simulink model "quantized"  (R2015b) (continued)
== system quantized == (continued)
  ri_to_c10/1 -> [ant_9]
  Convert20/1 -> Relational22/2, Mux20/2
  Relational22/1 -> Mux20/1
  Constant50/1 -> Mux20/3
  Constant43/1 -> Relational22/1
  Convert21/1 -> Relational23/2, Mux21/2
  Relational23/1 -> Mux21/1
  Constant51/1 -> Mux21/3
  Constant44/1 -> Relational23/1
  Mux20/1 -> ri_to_c11/1
  Mux21/1 -> ri_to_c11/2
  ri_to_c11/1 -> [ant_11]
  c_to_ri9/1 -> Mult22/1
  c_to_ri9/2 -> Mult23/2
  Constant52/1 -> scale8/1(sim_1)
  Mult22/1 -> Convert22/1
  Mult23/1 -> Convert23/1
  scale8/1(in_reg) -> Mult22/2, Mult23/1
  c_to_ri10/1 -> Mult16/1
  c_to_ri10/2 -> Mult17/2
  Constant53/1 -> scale10/1(sim_1)
  Mult16/1 -> Convert16/1
  Mult17/1 -> Convert17/1
  scale10/1(in_reg) -> Mult16/2, Mult17/1
  c_to_ri11/1 -> Mult18/1
  c_to_ri11/2 -> Mult19/2
  Constant54/1 -> scale9/1(sim_1)
  Mult18/1 -> Convert18/1
  Mult19/1 -> Convert19/1
  scale9/1(in_reg) -> Mult18/2, Mult19/1
  c_to_ri12/1 -> Mult20/1
  c_to_ri12/2 -> Mult21/2
  Constant55/1 -> scale11/1(sim_1)
  Mult20/1 -> Convert20/1
  Mult21/1 -> Convert21/1
  scale11/1(in_reg) -> Mult20/2, Mult21/1
  Register/1 -> c_to_ri1/1
  Register1/1 -> c_to_ri2/1
  Register2/1 -> c_to_ri3/1
  Register3/1 -> c_to_ri4/1
  Register4/1 -> c_to_ri5/1
  Register5/1 -> c_to_ri6/1
  Register6/1 -> c_to_ri7/1
  Register7/1 -> c_to_ri8/1
  Register8/1 -> c_to_ri9/1
  Register9/1 -> c_to_ri10/1
  Register10/1 -> c_to_ri11/1
  Register11/1 -> c_to_ri12/1
  addr_bram_vacc4/1(addr) -> ac_a4_real/1(addr)
  addr_bram_vacc4/2(dout) -> ac_a4_real/2(data_in)
  addr_bram_vacc4/3(we) -> ac_a4_real/3(we)
  ac_a4_real/1(data_out) -> Terminator8/1
  addr_bram_vacc5/1(addr) -> ac_a5_real/1(addr)
  addr_bram_vacc5/2(dout) -> ac_a5_real/2(data_in)
  addr_bram_vacc5/3(we) -> ac_a5_real/3(we)
  ac_a5_real/1(data_out) -> Terminator10/1
  addr_bram_vacc6/1(addr) -> ac_a6_real/1(addr)
  addr_bram_vacc6/2(dout) -> ac_a6_real/2(data_in)
  addr_bram_vacc6/3(we) -> ac_a6_real/3(we)
  ac_a6_real/1(data_out) -> Terminator7/1
  addr_bram_vacc7/1(addr) -> ac_a7_real/1(addr)
  addr_bram_vacc7/2(dout) -> ac_a7_real/2(data_in)
  addr_bram_vacc7/3(we) -> ac_a7_real/3(we)
  ac_a7_real/1(data_out) -> Terminator4/1
  [ant_4] -> cmult5/2(b), cmult5/1(a)
  [ant_5] -> cmult6/2(b), cmult6/1(a)
  [ant_6] -> cmult7/2(b), cmult7/1(a)
  [ant_7] -> cmult8/2(b), cmult8/1(a)
  Slice5/1 -> Reinterpret4/1
  Reinterpret4/1 -> addr_bram_vacc4/2(din)
  cmult5/1(ab) -> Slice5/1
  Slice6/1 -> Reinterpret5/1
  Reinterpret5/1 -> addr_bram_vacc5/2(din)
  cmult6/1(ab) -> Slice6/1
  Slice8/1 -> Reinterpret7/1
  Slice7/1 -> Reinterpret6/1
  Reinterpret6/1 -> addr_bram_vacc6/2(din)
  Reinterpret7/1 -> addr_bram_vacc7/2(din)
  cmult7/1(ab) -> Slice7/1
  cmult8/1(ab) -> Slice8/1
  addr_bram_vacc8/1(addr) -> ac_a8_real/1(addr)
  addr_bram_vacc8/2(dout) -> ac_a8_real/2(data_in)
  addr_bram_vacc8/3(we) -> ac_a8_real/3(we)
  ac_a8_real/1(data_out) -> Terminator14/1
  addr_bram_vacc9/1(addr) -> ac_a9_real/1(addr)
  addr_bram_vacc9/2(dout) -> ac_a9_real/2(data_in)
  addr_bram_vacc9/3(we) -> ac_a9_real/3(we)
  ac_a9_real/1(data_out) -> Terminator15/1
  [new_acc] -> addr_bram_vacc11/1(new_acc), addr_bram_vacc10/1(new_acc), addr_bram_vacc9/1(new_acc), addr_bram_vacc8/1(new_acc), addr_bram_vacc7/1(new_acc), addr_bram_vacc6/1(new_acc), addr_bram_vacc5/1(new_acc), addr_bram_vacc4/1(new_acc), addr_bram_vacc3/1(new_acc), addr_bram_vacc2/1(new_acc), addr_bram_vacc1/1(new_acc), addr_bram_vacc/1(new_acc)
  addr_bram_vacc10/1(addr) -> ac_a10_real/1(addr)
  addr_bram_vacc10/2(dout) -> ac_a10_real/2(data_in)
  addr_bram_vacc10/3(we) -> ac_a10_real/3(we)
  ac_a10_real/1(data_out) -> Terminator13/1
  addr_bram_vacc11/1(addr) -> ac_a11_real/1(addr)
  addr_bram_vacc11/2(dout) -> ac_a11_real/2(data_in)
  addr_bram_vacc11/3(we) -> ac_a11_real/3(we)
  ac_a11_real/1(data_out) -> Terminator12/1
  [ant_8] -> cmult9/2(b), cmult9/1(a)
  [ant_9] -> cmult10/2(b), cmult10/1(a)
  [ant_10] -> cmult11/2(b), cmult11/1(a)
  [ant_11] -> cmult12/2(b), cmult12/1(a)
  Slice9/1 -> Reinterpret8/1
  Reinterpret8/1 -> addr_bram_vacc8/2(din)
  cmult9/1(ab) -> Slice9/1
  Slice10/1 -> Reinterpret9/1
  Reinterpret9/1 -> addr_bram_vacc9/2(din)
  cmult10/1(ab) -> Slice10/1
  Slice12/1 -> Reinterpret11/1
  Slice11/1 -> Reinterpret10/1
  Reinterpret10/1 -> addr_bram_vacc10/2(din)
  Reinterpret11/1 -> addr_bram_vacc11/2(din)
  cmult11/1(ab) -> Slice11/1
  cmult12/1(ab) -> Slice12/1
  cmult/1(ab) -> c_to_ri/1
  addr_bram_vacc18/1(addr) -> cc_a0_a1_real/1(addr)
  addr_bram_vacc18/2(dout) -> cc_a0_a1_real/2(data_in)
  addr_bram_vacc18/3(we) -> cc_a0_a1_real/3(we)
  cc_a0_a1_real/1(data_out) -> Terminator26/1
  addr_bram_vacc19/1(addr) -> cc_a0_a1_imag/1(addr)
  addr_bram_vacc19/2(dout) -> cc_a0_a1_imag/2(data_in)
  addr_bram_vacc19/3(we) -> cc_a0_a1_imag/3(we)
  cc_a0_a1_imag/1(data_out) -> Terminator27/1
  c_to_ri/1 -> addr_bram_vacc18/2(din)
  c_to_ri/2 -> addr_bram_vacc19/2(din)
  cmult13/1(ab) -> c_to_ri75/1
  addr_bram_vacc20/1(addr) -> cc_a0_a2_real/1(addr)
  addr_bram_vacc20/2(dout) -> cc_a0_a2_real/2(data_in)
  addr_bram_vacc20/3(we) -> cc_a0_a2_real/3(we)
  cc_a0_a2_real/1(data_out) -> Terminator16/1
  addr_bram_vacc21/1(addr) -> cc_a0_a2_imag/1(addr)
  addr_bram_vacc21/2(dout) -> cc_a0_a2_imag/2(data_in)
  addr_bram_vacc21/3(we) -> cc_a0_a2_imag/3(we)
  cc_a0_a2_imag/1(data_out) -> Terminator17/1
  c_to_ri75/1 -> addr_bram_vacc20/2(din)
  c_to_ri75/2 -> addr_bram_vacc21/2(din)
  cmult14/1(ab) -> c_to_ri76/1
  addr_bram_vacc22/1(addr) -> cc_a0_a3_real/1(addr)
  addr_bram_vacc22/2(dout) -> cc_a0_a3_real/2(data_in)
  addr_bram_vacc22/3(we) -> cc_a0_a3_real/3(we)
  cc_a0_a3_real/1(data_out) -> Terminator18/1
  addr_bram_vacc23/1(addr) -> cc_a0_a3_imag/1(addr)
  addr_bram_vacc23/2(dout) -> cc_a0_a3_imag/2(data_in)
  addr_bram_vacc23/3(we) -> cc_a0_a3_imag/3(we)
  cc_a0_a3_imag/1(data_out) -> Terminator19/1
  c_to_ri76/1 -> addr_bram_vacc22/2(din)
  c_to_ri76/2 -> addr_bram_vacc23/2(din)
  cmult15/1(ab) -> c_to_ri77/1
  addr_bram_vacc12/1(addr) -> cc_a1_a2_real/1(addr)
  addr_bram_vacc12/2(dout) -> cc_a1_a2_real/2(data_in)
  addr_bram_vacc12/3(we) -> cc_a1_a2_real/3(we)
  cc_a1_a2_real/1(data_out) -> Terminator20/1
  addr_bram_vacc13/1(addr) -> cc_a1_a2_imag/1(addr)
  addr_bram_vacc13/2(dout) -> cc_a1_a2_imag/2(data_in)
  addr_bram_vacc13/3(we) -> cc_a1_a2_imag/3(we)
  cc_a1_a2_imag/1(data_out) -> Terminator21/1
  c_to_ri77/1 -> addr_bram_vacc12/2(din)
  c_to_ri77/2 -> addr_bram_vacc13/2(din)
  cmult16/1(ab) -> c_to_ri14/1
  addr_bram_vacc14/1(addr) -> cc_a1_a3_real/1(addr)
  addr_bram_vacc14/2(dout) -> cc_a1_a3_real/2(data_in)
  addr_bram_vacc14/3(we) -> cc_a1_a3_real/3(we)
  cc_a1_a3_real/1(data_out) -> Terminator22/1
  addr_bram_vacc15/1(addr) -> cc_a1_a3_imag/1(addr)
  addr_bram_vacc15/2(dout) -> cc_a1_a3_imag/2(data_in)
  addr_bram_vacc15/3(we) -> cc_a1_a3_imag/3(we)
  cc_a1_a3_imag/1(data_out) -> Terminator23/1
  c_to_ri14/1 -> addr_bram_vacc14/2(din)
  c_to_ri14/2 -> addr_bram_vacc15/2(din)
  [ant_0] -> cmult/1(a)
  [ant_1] -> cmult/2(b)
  [ant_0] -> cmult13/1(a)
  [ant_2] -> cmult13/2(b)
  [ant_3] -> cmult14/2(b)
  [ant_0] -> cmult14/1(a)
  [ant_2] -> cmult15/2(b)
  [ant_1] -> cmult15/1(a)
  [ant_3] -> cmult16/2(b)
  [ant_1] -> cmult16/1(a)
  cmult41/1(ab) -> c_to_ri36/1
  addr_bram_vacc16/1(addr) -> cc_a2_a3_real/1(addr)
  addr_bram_vacc16/2(dout) -> cc_a2_a3_real/2(data_in)
  addr_bram_vacc16/3(we) -> cc_a2_a3_real/3(we)
  cc_a2_a3_real/1(data_out) -> Terminator24/1
  addr_bram_vacc17/1(addr) -> cc_a2_a3_imag/1(addr)
  addr_bram_vacc17/2(dout) -> cc_a2_a3_imag/2(data_in)
  addr_bram_vacc17/3(we) -> cc_a2_a3_imag/3(we)
  cc_a2_a3_imag/1(data_out) -> Terminator25/1
  c_to_ri36/1 -> addr_bram_vacc16/2(din)
  c_to_ri36/2 -> addr_bram_vacc17/2(din)
  [ant_3] -> cmult41/2(b)
  [ant_2] -> cmult41/1(a)
  cmult17/1(ab) -> c_to_ri13/1
  addr_bram_vacc24/1(addr) -> cc_a0_a4_real/1(addr)
  addr_bram_vacc24/2(dout) -> cc_a0_a4_real/2(data_in)
  addr_bram_vacc24/3(we) -> cc_a0_a4_real/3(we)
  cc_a0_a4_real/1(data_out) -> Terminator32/1
  addr_bram_vacc25/1(addr) -> cc_a0_a4_imag/1(addr)
  addr_bram_vacc25/2(dout) -> cc_a0_a4_imag/2(data_in)
  addr_bram_vacc25/3(we) -> cc_a0_a4_imag/3(we)
  cc_a0_a4_imag/1(data_out) -> Terminator33/1
  c_to_ri13/1 -> addr_bram_vacc24/2(din)
  c_to_ri13/2 -> addr_bram_vacc25/2(din)
  cmult18/1(ab) -> c_to_ri24/1
  addr_bram_vacc26/1(addr) -> cc_a0_a5_real/1(addr)
  addr_bram_vacc26/2(dout) -> cc_a0_a5_real/2(data_in)
  addr_bram_vacc26/3(we) -> cc_a0_a5_real/3(we)
  cc_a0_a5_real/1(data_out) -> Terminator28/1
  addr_bram_vacc27/1(addr) -> cc_a0_a5_imag/1(addr)
  addr_bram_vacc27/2(dout) -> cc_a0_a5_imag/2(data_in)
  addr_bram_vacc27/3(we) -> cc_a0_a5_imag/3(we)
  cc_a0_a5_imag/1(data_out) -> Terminator29/1
  c_to_ri24/1 -> addr_bram_vacc26/2(din)
  c_to_ri24/2 -> addr_bram_vacc27/2(din)
  cmult19/1(ab) -> c_to_ri35/1
  addr_bram_vacc28/1(addr) -> cc_a0_a6_real/1(addr)
  addr_bram_vacc28/2(dout) -> cc_a0_a6_real/2(data_in)
  addr_bram_vacc28/3(we) -> cc_a0_a6_real/3(we)
  cc_a0_a6_real/1(data_out) -> Terminator30/1
  addr_bram_vacc29/1(addr) -> cc_a0_a6_imag/1(addr)
  addr_bram_vacc29/2(dout) -> cc_a0_a6_imag/2(data_in)
  addr_bram_vacc29/3(we) -> cc_a0_a6_imag/3(we)
  cc_a0_a6_imag/1(data_out) -> Terminator31/1
  c_to_ri35/1 -> addr_bram_vacc28/2(din)
  c_to_ri35/2 -> addr_bram_vacc29/2(din)
  [ant_0] -> cmult17/1(a)
  [ant_4] -> cmult17/2(b)
  [ant_0] -> cmult18/1(a)
  [ant_5] -> cmult18/2(b)
  [ant_6] -> cmult19/2(b)
  [ant_0] -> cmult19/1(a)
  cmult20/1(ab) -> c_to_ri46/1
  addr_bram_vacc30/1(addr) -> cc_a0_a7_real/1(addr)
  addr_bram_vacc30/2(dout) -> cc_a0_a7_real/2(data_in)
  addr_bram_vacc30/3(we) -> cc_a0_a7_real/3(we)
  cc_a0_a7_real/1(data_out) -> Terminator38/1
  addr_bram_vacc31/1(addr) -> cc_a0_a7_imag/1(addr)
  addr_bram_vacc31/2(dout) -> cc_a0_a7_imag/2(data_in)
  addr_bram_vacc31/3(we) -> cc_a0_a7_imag/3(we)
  cc_a0_a7_imag/1(data_out) -> Terminator39/1
  c_to_ri46/1 -> addr_bram_vacc30/2(din)
  c_to_ri46/2 -> addr_bram_vacc31/2(din)
  cmult21/1(ab) -> c_to_ri57/1
  addr_bram_vacc32/1(addr) -> cc_a0_a8_real/1(addr)
  addr_bram_vacc32/2(dout) -> cc_a0_a8_real/2(data_in)
  addr_bram_vacc32/3(we) -> cc_a0_a8_real/3(we)
  cc_a0_a8_real/1(data_out) -> Terminator34/1
  addr_bram_vacc33/1(addr) -> cc_a0_a8_imag/1(addr)
  addr_bram_vacc33/2(dout) -> cc_a0_a8_imag/2(data_in)
  addr_bram_vacc33/3(we) -> cc_a0_a8_imag/3(we)
  cc_a0_a8_imag/1(data_out) -> Terminator35/1
  c_to_ri57/1 -> addr_bram_vacc32/2(din)
  c_to_ri57/2 -> addr_bram_vacc33/2(din)
  cmult22/1(ab) -> c_to_ri68/1
  addr_bram_vacc34/1(addr) -> cc_a0_a9_real/1(addr)
  addr_bram_vacc34/2(dout) -> cc_a0_a9_real/2(data_in)
  addr_bram_vacc34/3(we) -> cc_a0_a9_real/3(we)
  cc_a0_a9_real/1(data_out) -> Terminator36/1
  addr_bram_vacc35/1(addr) -> cc_a0_a9_imag/1(addr)
  addr_bram_vacc35/2(dout) -> cc_a0_a9_imag/2(data_in)
  addr_bram_vacc35/3(we) -> cc_a0_a9_imag/3(we)
  cc_a0_a9_imag/1(data_out) -> Terminator37/1
  c_to_ri68/1 -> addr_bram_vacc34/2(din)
  c_to_ri68/2 -> addr_bram_vacc35/2(din)
  [ant_0] -> cmult20/1(a)
  [ant_7] -> cmult20/2(b)
  [ant_0] -> cmult21/1(a)
  [ant_8] -> cmult21/2(b)
  [ant_9] -> cmult22/2(b)
  [ant_0] -> cmult22/1(a)
  cmult23/1(ab) -> c_to_ri15/1
  addr_bram_vacc36/1(addr) -> cc_a0_a10_real/1(addr)
  addr_bram_vacc36/2(dout) -> cc_a0_a10_real/2(data_in)
  addr_bram_vacc36/3(we) -> cc_a0_a10_real/3(we)
  cc_a0_a10_real/1(data_out) -> Terminator44/1
  addr_bram_vacc37/1(addr) -> cc_a0_a10_imag/1(addr)
  addr_bram_vacc37/2(dout) -> cc_a0_a10_imag/2(data_in)
  addr_bram_vacc37/3(we) -> cc_a0_a10_imag/3(we)
  cc_a0_a10_imag/1(data_out) -> Terminator45/1
  c_to_ri15/1 -> addr_bram_vacc36/2(din)
  c_to_ri15/2 -> addr_bram_vacc37/2(din)
  cmult24/1(ab) -> c_to_ri16/1
  addr_bram_vacc38/1(addr) -> cc_a0_a11_real/1(addr)
  addr_bram_vacc38/2(dout) -> cc_a0_a11_real/2(data_in)
  addr_bram_vacc38/3(we) -> cc_a0_a11_real/3(we)
  cc_a0_a11_real/1(data_out) -> Terminator40/1
  addr_bram_vacc39/1(addr) -> cc_a0_a11_imag/1(addr)
  addr_bram_vacc39/2(dout) -> cc_a0_a11_imag/2(data_in)
  addr_bram_vacc39/3(we) -> cc_a0_a11_imag/3(we)
  cc_a0_a11_imag/1(data_out) -> Terminator41/1
  c_to_ri16/1 -> addr_bram_vacc38/2(din)
  c_to_ri16/2 -> addr_bram_vacc39/2(din)
  [ant_0] -> cmult23/1(a)
  [ant_10] -> cmult23/2(b)
  [ant_0] -> cmult24/1(a)
  [ant_11] -> cmult24/2(b)
  [new_acc] -> addr_bram_vacc19/1(new_acc), addr_bram_vacc20/1(new_acc), addr_bram_vacc23/1(new_acc), addr_bram_vacc24/1(new_acc), addr_bram_vacc25/1(new_acc), addr_bram_vacc26/1(new_acc), addr_bram_vacc30/1(new_acc), addr_bram_vacc31/1(new_acc), addr_bram_vacc32/1(new_acc), addr_bram_vacc35/1(new_acc), addr_bram_vacc36/1(new_acc), addr_bram_vacc37/1(new_acc), addr_bram_vacc38/1(new_acc), addr_bram_vacc39/1(new_acc), addr_bram_vacc34/1(new_acc), addr_bram_vacc33/1(new_acc), addr_bram_vacc29/1(new_acc), addr_bram_vacc28/1(new_acc), addr_bram_vacc27/1(new_acc), addr_bram_vacc22/1(new_acc), addr_bram_vacc21/1(new_acc), addr_bram_vacc18/1(new_acc)
  cmult25/1(ab) -> c_to_ri18/1
  addr_bram_vacc40/1(addr) -> cc_a1_a4_real/1(addr)
  addr_bram_vacc40/2(dout) -> cc_a1_a4_real/2(data_in)
  addr_bram_vacc40/3(we) -> cc_a1_a4_real/3(we)
  cc_a1_a4_real/1(data_out) -> Terminator42/1
  addr_bram_vacc41/1(addr) -> cc_a1_a4_imag/1(addr)
  addr_bram_vacc41/2(dout) -> cc_a1_a4_imag/2(data_in)
  addr_bram_vacc41/3(we) -> cc_a1_a4_imag/3(we)
  cc_a1_a4_imag/1(data_out) -> Terminator43/1
  c_to_ri18/1 -> addr_bram_vacc40/2(din)
  c_to_ri18/2 -> addr_bram_vacc41/2(din)
  cmult26/1(ab) -> c_to_ri17/1
  addr_bram_vacc42/1(addr) -> cc_a1_a5_real/1(addr)
  addr_bram_vacc42/2(dout) -> cc_a1_a5_real/2(data_in)
  addr_bram_vacc42/3(we) -> cc_a1_a5_real/3(we)
  cc_a1_a5_real/1(data_out) -> Terminator46/1
  addr_bram_vacc43/1(addr) -> cc_a1_a5_imag/1(addr)
  addr_bram_vacc43/2(dout) -> cc_a1_a5_imag/2(data_in)
  addr_bram_vacc43/3(we) -> cc_a1_a5_imag/3(we)
  cc_a1_a5_imag/1(data_out) -> Terminator47/1
  c_to_ri17/1 -> addr_bram_vacc42/2(din)
  c_to_ri17/2 -> addr_bram_vacc43/2(din)
  [ant_4] -> cmult25/2(b)
  [ant_1] -> cmult25/1(a)
  [ant_5] -> cmult26/2(b)
  [ant_1] -> cmult26/1(a)
  cmult27/1(ab) -> c_to_ri20/1
  addr_bram_vacc44/1(addr) -> cc_a1_a6_real/1(addr)
  addr_bram_vacc44/2(dout) -> cc_a1_a6_real/2(data_in)
  addr_bram_vacc44/3(we) -> cc_a1_a6_real/3(we)
  cc_a1_a6_real/1(data_out) -> Terminator48/1
  addr_bram_vacc45/1(addr) -> cc_a1_a6_imag/1(addr)
  addr_bram_vacc45/2(dout) -> cc_a1_a6_imag/2(data_in)
  addr_bram_vacc45/3(we) -> cc_a1_a6_imag/3(we)
  cc_a1_a6_imag/1(data_out) -> Terminator49/1
  c_to_ri20/1 -> addr_bram_vacc44/2(din)
  c_to_ri20/2 -> addr_bram_vacc45/2(din)
  cmult28/1(ab) -> c_to_ri19/1
  addr_bram_vacc46/1(addr) -> cc_a1_a7_real/1(addr)
  addr_bram_vacc46/2(dout) -> cc_a1_a7_real/2(data_in)
  addr_bram_vacc46/3(we) -> cc_a1_a7_real/3(we)
  cc_a1_a7_real/1(data_out) -> Terminator50/1
  addr_bram_vacc47/1(addr) -> cc_a1_a7_imag/1(addr)
  addr_bram_vacc47/2(dout) -> cc_a1_a7_imag/2(data_in)
  addr_bram_vacc47/3(we) -> cc_a1_a7_imag/3(we)
  cc_a1_a7_imag/1(data_out) -> Terminator51/1
  c_to_ri19/1 -> addr_bram_vacc46/2(din)
  c_to_ri19/2 -> addr_bram_vacc47/2(din)
  [ant_6] -> cmult27/2(b)
  [ant_1] -> cmult27/1(a)
  [ant_7] -> cmult28/2(b)
  [ant_1] -> cmult28/1(a)
  cmult29/1(ab) -> c_to_ri22/1
  addr_bram_vacc48/1(addr) -> cc_a1_a8_real/1(addr)
  addr_bram_vacc48/2(dout) -> cc_a1_a8_real/2(data_in)
  addr_bram_vacc48/3(we) -> cc_a1_a8_real/3(we)
  cc_a1_a8_real/1(data_out) -> Terminator52/1
  addr_bram_vacc49/1(addr) -> cc_a1_a8_imag/1(addr)
  addr_bram_vacc49/2(dout) -> cc_a1_a8_imag/2(data_in)
  addr_bram_vacc49/3(we) -> cc_a1_a8_imag/3(we)
  cc_a1_a8_imag/1(data_out) -> Terminator53/1
  c_to_ri22/1 -> addr_bram_vacc48/2(din)
  c_to_ri22/2 -> addr_bram_vacc49/2(din)
  cmult30/1(ab) -> c_to_ri21/1
  addr_bram_vacc50/1(addr) -> cc_a1_a9_real/1(addr)
  addr_bram_vacc50/2(dout) -> cc_a1_a9_real/2(data_in)
  addr_bram_vacc50/3(we) -> cc_a1_a9_real/3(we)
  cc_a1_a9_real/1(data_out) -> Terminator54/1
  addr_bram_vacc51/1(addr) -> cc_a1_a9_imag/1(addr)
  addr_bram_vacc51/2(dout) -> cc_a1_a9_imag/2(data_in)
  addr_bram_vacc51/3(we) -> cc_a1_a9_imag/3(we)
  cc_a1_a9_imag/1(data_out) -> Terminator55/1
  c_to_ri21/1 -> addr_bram_vacc50/2(din)
  c_to_ri21/2 -> addr_bram_vacc51/2(din)
  [ant_8] -> cmult29/2(b)
  [ant_1] -> cmult29/1(a)
  [ant_9] -> cmult30/2(b)
  [ant_1] -> cmult30/1(a)
  cmult31/1(ab) -> c_to_ri25/1
  addr_bram_vacc52/1(addr) -> cc_a1_a10_real/1(addr)
  addr_bram_vacc52/2(dout) -> cc_a1_a10_real/2(data_in)
  addr_bram_vacc52/3(we) -> cc_a1_a10_real/3(we)
  cc_a1_a10_real/1(data_out) -> Terminator56/1
  addr_bram_vacc53/1(addr) -> cc_a1_a10_imag/1(addr)
  addr_bram_vacc53/2(dout) -> cc_a1_a10_imag/2(data_in)
  addr_bram_vacc53/3(we) -> cc_a1_a10_imag/3(we)
  cc_a1_a10_imag/1(data_out) -> Terminator57/1
  c_to_ri25/1 -> addr_bram_vacc52/2(din)
  c_to_ri25/2 -> addr_bram_vacc53/2(din)
  cmult32/1(ab) -> c_to_ri23/1
  addr_bram_vacc54/1(addr) -> cc_a1_a11_real/1(addr)
  addr_bram_vacc54/2(dout) -> cc_a1_a11_real/2(data_in)
  addr_bram_vacc54/3(we) -> cc_a1_a11_real/3(we)
  cc_a1_a11_real/1(data_out) -> Terminator58/1
  addr_bram_vacc55/1(addr) -> cc_a1_a11_imag/1(addr)
  addr_bram_vacc55/2(dout) -> cc_a1_a11_imag/2(data_in)
  addr_bram_vacc55/3(we) -> cc_a1_a11_imag/3(we)
  cc_a1_a11_imag/1(data_out) -> Terminator59/1
  c_to_ri23/1 -> addr_bram_vacc54/2(din)
  c_to_ri23/2 -> addr_bram_vacc55/2(din)
  [ant_10] -> cmult31/2(b)
  [ant_1] -> cmult31/1(a)
  [ant_11] -> cmult32/2(b)
  [ant_1] -> cmult32/1(a)
  [new_acc] -> addr_bram_vacc55/1(new_acc), addr_bram_vacc54/1(new_acc), addr_bram_vacc53/1(new_acc), addr_bram_vacc52/1(new_acc), addr_bram_vacc51/1(new_acc), addr_bram_vacc50/1(new_acc), addr_bram_vacc49/1(new_acc), addr_bram_vacc48/1(new_acc), addr_bram_vacc47/1(new_acc), addr_bram_vacc46/1(new_acc), addr_bram_vacc45/1(new_acc), addr_bram_vacc44/1(new_acc), addr_bram_vacc43/1(new_acc), addr_bram_vacc42/1(new_acc), addr_bram_vacc41/1(new_acc), addr_bram_vacc40/1(new_acc), addr_bram_vacc15/1(new_acc), addr_bram_vacc14/1(new_acc), addr_bram_vacc13/1(new_acc), addr_bram_vacc12/1(new_acc)
  cmult33/1(ab) -> c_to_ri26/1
  addr_bram_vacc56/1(addr) -> cc_a2_a4_real/1(addr)
  addr_bram_vacc56/2(dout) -> cc_a2_a4_real/2(data_in)
  addr_bram_vacc56/3(we) -> cc_a2_a4_real/3(we)
  cc_a2_a4_real/1(data_out) -> Terminator60/1
  addr_bram_vacc57/1(addr) -> cc_a2_a4_imag/1(addr)
  addr_bram_vacc57/2(dout) -> cc_a2_a4_imag/2(data_in)
  addr_bram_vacc57/3(we) -> cc_a2_a4_imag/3(we)
  cc_a2_a4_imag/1(data_out) -> Terminator61/1
  c_to_ri26/1 -> addr_bram_vacc56/2(din)
  c_to_ri26/2 -> addr_bram_vacc57/2(din)
  [ant_4] -> cmult33/2(b)
  [ant_2] -> cmult33/1(a)
  cmult34/1(ab) -> c_to_ri27/1
  addr_bram_vacc58/1(addr) -> cc_a2_a5_real/1(addr)
  addr_bram_vacc58/2(dout) -> cc_a2_a5_real/2(data_in)
  addr_bram_vacc58/3(we) -> cc_a2_a5_real/3(we)
  cc_a2_a5_real/1(data_out) -> Terminator62/1
  addr_bram_vacc59/1(addr) -> cc_a2_a5_imag/1(addr)
  addr_bram_vacc59/2(dout) -> cc_a2_a5_imag/2(data_in)
  addr_bram_vacc59/3(we) -> cc_a2_a5_imag/3(we)
  cc_a2_a5_imag/1(data_out) -> Terminator63/1
  c_to_ri27/1 -> addr_bram_vacc58/2(din)
  c_to_ri27/2 -> addr_bram_vacc59/2(din)
  [ant_5] -> cmult34/2(b)
  [ant_2] -> cmult34/1(a)
  cmult35/1(ab) -> c_to_ri28/1
  addr_bram_vacc60/1(addr) -> cc_a2_a6_real/1(addr)
  addr_bram_vacc60/2(dout) -> cc_a2_a6_real/2(data_in)
  addr_bram_vacc60/3(we) -> cc_a2_a6_real/3(we)
  cc_a2_a6_real/1(data_out) -> Terminator64/1
  addr_bram_vacc61/1(addr) -> cc_a2_a6_imag/1(addr)
  addr_bram_vacc61/2(dout) -> cc_a2_a6_imag/2(data_in)
  addr_bram_vacc61/3(we) -> cc_a2_a6_imag/3(we)
  cc_a2_a6_imag/1(data_out) -> Terminator65/1
  c_to_ri28/1 -> addr_bram_vacc60/2(din)
  c_to_ri28/2 -> addr_bram_vacc61/2(din)
  [ant_6] -> cmult35/2(b)
  [ant_2] -> cmult35/1(a)
  cmult36/1(ab) -> c_to_ri29/1
  addr_bram_vacc62/1(addr) -> cc_a2_a7_real/1(addr)
  addr_bram_vacc62/2(dout) -> cc_a2_a7_real/2(data_in)
  addr_bram_vacc62/3(we) -> cc_a2_a7_real/3(we)
  cc_a2_a7_real/1(data_out) -> Terminator66/1
  addr_bram_vacc63/1(addr) -> cc_a2_a7_imag/1(addr)
  addr_bram_vacc63/2(dout) -> cc_a2_a7_imag/2(data_in)
  addr_bram_vacc63/3(we) -> cc_a2_a7_imag/3(we)
  cc_a2_a7_imag/1(data_out) -> Terminator67/1
  c_to_ri29/1 -> addr_bram_vacc62/2(din)
  c_to_ri29/2 -> addr_bram_vacc63/2(din)
  [ant_7] -> cmult36/2(b)
  [ant_2] -> cmult36/1(a)
  cmult37/1(ab) -> c_to_ri30/1
  addr_bram_vacc64/1(addr) -> cc_a2_a8_real/1(addr)
  addr_bram_vacc64/2(dout) -> cc_a2_a8_real/2(data_in)
  addr_bram_vacc64/3(we) -> cc_a2_a8_real/3(we)
  cc_a2_a8_real/1(data_out) -> Terminator68/1
  addr_bram_vacc65/1(addr) -> cc_a2_a8_imag/1(addr)
  addr_bram_vacc65/2(dout) -> cc_a2_a8_imag/2(data_in)
  addr_bram_vacc65/3(we) -> cc_a2_a8_imag/3(we)
  cc_a2_a8_imag/1(data_out) -> Terminator69/1
  c_to_ri30/1 -> addr_bram_vacc64/2(din)
  c_to_ri30/2 -> addr_bram_vacc65/2(din)
  [ant_8] -> cmult37/2(b)
  [ant_2] -> cmult37/1(a)
  cmult38/1(ab) -> c_to_ri31/1
  addr_bram_vacc66/1(addr) -> cc_a2_a9_real/1(addr)
  addr_bram_vacc66/2(dout) -> cc_a2_a9_real/2(data_in)
  addr_bram_vacc66/3(we) -> cc_a2_a9_real/3(we)
  cc_a2_a9_real/1(data_out) -> Terminator70/1
  addr_bram_vacc67/1(addr) -> cc_a2_a9_imag/1(addr)
  addr_bram_vacc67/2(dout) -> cc_a2_a9_imag/2(data_in)
  addr_bram_vacc67/3(we) -> cc_a2_a9_imag/3(we)
  cc_a2_a9_imag/1(data_out) -> Terminator71/1
  c_to_ri31/1 -> addr_bram_vacc66/2(din)
  c_to_ri31/2 -> addr_bram_vacc67/2(din)
  [ant_9] -> cmult38/2(b)
  [ant_2] -> cmult38/1(a)
  cmult39/1(ab) -> c_to_ri32/1
  addr_bram_vacc68/1(addr) -> cc_a2_a10_real/1(addr)
  addr_bram_vacc68/2(dout) -> cc_a2_a10_real/2(data_in)
  addr_bram_vacc68/3(we) -> cc_a2_a10_real/3(we)
  cc_a2_a10_real/1(data_out) -> Terminator72/1
  addr_bram_vacc69/1(addr) -> cc_a2_a10_imag/1(addr)
  addr_bram_vacc69/2(dout) -> cc_a2_a10_imag/2(data_in)
  addr_bram_vacc69/3(we) -> cc_a2_a10_imag/3(we)
  cc_a2_a10_imag/1(data_out) -> Terminator73/1
  c_to_ri32/1 -> addr_bram_vacc68/2(din)
  c_to_ri32/2 -> addr_bram_vacc69/2(din)
  [ant_10] -> cmult39/2(b)
  [ant_2] -> cmult39/1(a)
  cmult40/1(ab) -> c_to_ri33/1
  addr_bram_vacc70/1(addr) -> cc_a2_a11_real/1(addr)
  addr_bram_vacc70/2(dout) -> cc_a2_a11_real/2(data_in)
  addr_bram_vacc70/3(we) -> cc_a2_a11_real/3(we)
  cc_a2_a11_real/1(data_out) -> Terminator74/1
  addr_bram_vacc71/1(addr) -> cc_a2_a11_imag/1(addr)
  addr_bram_vacc71/2(dout) -> cc_a2_a11_imag/2(data_in)
  addr_bram_vacc71/3(we) -> cc_a2_a11_imag/3(we)
  cc_a2_a11_imag/1(data_out) -> Terminator75/1
  c_to_ri33/1 -> addr_bram_vacc70/2(din)
  c_to_ri33/2 -> addr_bram_vacc71/2(din)
  [ant_11] -> cmult40/2(b)
  [ant_2] -> cmult40/1(a)
  [new_acc] -> addr_bram_vacc61/1(new_acc), addr_bram_vacc62/1(new_acc), addr_bram_vacc71/1(new_acc), addr_bram_vacc70/1(new_acc), addr_bram_vacc69/1(new_acc), addr_bram_vacc68/1(new_acc), addr_bram_vacc67/1(new_acc), addr_bram_vacc66/1(new_acc), addr_bram_vacc65/1(new_acc), addr_bram_vacc64/1(new_acc), addr_bram_vacc63/1(new_acc), addr_bram_vacc60/1(new_acc), addr_bram_vacc59/1(new_acc), addr_bram_vacc58/1(new_acc), addr_bram_vacc57/1(new_acc), addr_bram_vacc56/1(new_acc), addr_bram_vacc17/1(new_acc), addr_bram_vacc16/1(new_acc)
  cmult42/1(ab) -> c_to_ri34/1
  addr_bram_vacc72/1(addr) -> cc_a3_a4_real/1(addr)
  addr_bram_vacc72/2(dout) -> cc_a3_a4_real/2(data_in)
  addr_bram_vacc72/3(we) -> cc_a3_a4_real/3(we)
  cc_a3_a4_real/1(data_out) -> Terminator76/1
  addr_bram_vacc73/1(addr) -> cc_a3_a4_imag/1(addr)
  addr_bram_vacc73/2(dout) -> cc_a3_a4_imag/2(data_in)
  addr_bram_vacc73/3(we) -> cc_a3_a4_imag/3(we)
  cc_a3_a4_imag/1(data_out) -> Terminator77/1
  c_to_ri34/1 -> addr_bram_vacc72/2(din)
  c_to_ri34/2 -> addr_bram_vacc73/2(din)
  [ant_4] -> cmult42/2(b)
  [ant_3] -> cmult42/1(a)
  cmult43/1(ab) -> c_to_ri37/1
  addr_bram_vacc74/1(addr) -> cc_a3_a5_real/1(addr)
  addr_bram_vacc74/2(dout) -> cc_a3_a5_real/2(data_in)
  addr_bram_vacc74/3(we) -> cc_a3_a5_real/3(we)
  cc_a3_a5_real/1(data_out) -> Terminator78/1
  addr_bram_vacc75/1(addr) -> cc_a3_a5_imag/1(addr)
  addr_bram_vacc75/2(dout) -> cc_a3_a5_imag/2(data_in)
  addr_bram_vacc75/3(we) -> cc_a3_a5_imag/3(we)
  cc_a3_a5_imag/1(data_out) -> Terminator79/1
  c_to_ri37/1 -> addr_bram_vacc74/2(din)
  c_to_ri37/2 -> addr_bram_vacc75/2(din)
  [ant_5] -> cmult43/2(b)
  [ant_3] -> cmult43/1(a)
  cmult44/1(ab) -> c_to_ri38/1
  addr_bram_vacc76/1(addr) -> cc_a3_a6_real/1(addr)
  addr_bram_vacc76/2(dout) -> cc_a3_a6_real/2(data_in)
  addr_bram_vacc76/3(we) -> cc_a3_a6_real/3(we)
  cc_a3_a6_real/1(data_out) -> Terminator80/1
  addr_bram_vacc77/1(addr) -> cc_a3_a6_imag/1(addr)
  addr_bram_vacc77/2(dout) -> cc_a3_a6_imag/2(data_in)
  addr_bram_vacc77/3(we) -> cc_a3_a6_imag/3(we)
  cc_a3_a6_imag/1(data_out) -> Terminator81/1
  c_to_ri38/1 -> addr_bram_vacc76/2(din)
  c_to_ri38/2 -> addr_bram_vacc77/2(din)
  [ant_6] -> cmult44/2(b)
  [ant_3] -> cmult44/1(a)
  cmult45/1(ab) -> c_to_ri39/1
  addr_bram_vacc78/1(addr) -> cc_a3_a7_real/1(addr)
  addr_bram_vacc78/2(dout) -> cc_a3_a7_real/2(data_in)
  addr_bram_vacc78/3(we) -> cc_a3_a7_real/3(we)
  cc_a3_a7_real/1(data_out) -> Terminator82/1
  addr_bram_vacc79/1(addr) -> cc_a3_a7_imag/1(addr)
  addr_bram_vacc79/2(dout) -> cc_a3_a7_imag/2(data_in)
  addr_bram_vacc79/3(we) -> cc_a3_a7_imag/3(we)
  cc_a3_a7_imag/1(data_out) -> Terminator83/1
  c_to_ri39/1 -> addr_bram_vacc78/2(din)
  c_to_ri39/2 -> addr_bram_vacc79/2(din)
  [ant_7] -> cmult45/2(b)
  [ant_3] -> cmult45/1(a)
  cmult46/1(ab) -> c_to_ri40/1
  addr_bram_vacc80/1(addr) -> cc_a3_a8_real/1(addr)
  addr_bram_vacc80/2(dout) -> cc_a3_a8_real/2(data_in)
  addr_bram_vacc80/3(we) -> cc_a3_a8_real/3(we)
  cc_a3_a8_real/1(data_out) -> Terminator84/1
  addr_bram_vacc81/1(addr) -> cc_a3_a8_imag/1(addr)
  addr_bram_vacc81/2(dout) -> cc_a3_a8_imag/2(data_in)
  addr_bram_vacc81/3(we) -> cc_a3_a8_imag/3(we)
  cc_a3_a8_imag/1(data_out) -> Terminator85/1
  c_to_ri40/1 -> addr_bram_vacc80/2(din)
  c_to_ri40/2 -> addr_bram_vacc81/2(din)
  [ant_8] -> cmult46/2(b)
  [ant_3] -> cmult46/1(a)
  cmult47/1(ab) -> c_to_ri41/1
  addr_bram_vacc82/1(addr) -> cc_a3_a9_real/1(addr)
  addr_bram_vacc82/2(dout) -> cc_a3_a9_real/2(data_in)
  addr_bram_vacc82/3(we) -> cc_a3_a9_real/3(we)
  cc_a3_a9_real/1(data_out) -> Terminator86/1
  addr_bram_vacc83/1(addr) -> cc_a3_a9_imag/1(addr)
  addr_bram_vacc83/2(dout) -> cc_a3_a9_imag/2(data_in)
  addr_bram_vacc83/3(we) -> cc_a3_a9_imag/3(we)
  cc_a3_a9_imag/1(data_out) -> Terminator87/1
  c_to_ri41/1 -> addr_bram_vacc82/2(din)
  c_to_ri41/2 -> addr_bram_vacc83/2(din)
  [ant_9] -> cmult47/2(b)
  [ant_3] -> cmult47/1(a)
  cmult48/1(ab) -> c_to_ri42/1
  addr_bram_vacc84/1(addr) -> cc_a3_a10_real/1(addr)
  addr_bram_vacc84/2(dout) -> cc_a3_a10_real/2(data_in)
  addr_bram_vacc84/3(we) -> cc_a3_a10_real/3(we)
  cc_a3_a10_real/1(data_out) -> Terminator88/1
  addr_bram_vacc85/1(addr) -> cc_a3_a10_imag/1(addr)
  addr_bram_vacc85/2(dout) -> cc_a3_a10_imag/2(data_in)
  addr_bram_vacc85/3(we) -> cc_a3_a10_imag/3(we)
  cc_a3_a10_imag/1(data_out) -> Terminator89/1
  c_to_ri42/1 -> addr_bram_vacc84/2(din)
  c_to_ri42/2 -> addr_bram_vacc85/2(din)
  [ant_10] -> cmult48/2(b)
  [ant_3] -> cmult48/1(a)
  cmult49/1(ab) -> c_to_ri43/1
  addr_bram_vacc86/1(addr) -> cc_a3_a11_real/1(addr)
  addr_bram_vacc86/2(dout) -> cc_a3_a11_real/2(data_in)
  addr_bram_vacc86/3(we) -> cc_a3_a11_real/3(we)
  cc_a3_a11_real/1(data_out) -> Terminator90/1
  addr_bram_vacc87/1(addr) -> cc_a3_a11_imag/1(addr)
  addr_bram_vacc87/2(dout) -> cc_a3_a11_imag/2(data_in)
  addr_bram_vacc87/3(we) -> cc_a3_a11_imag/3(we)
  cc_a3_a11_imag/1(data_out) -> Terminator91/1
  c_to_ri43/1 -> addr_bram_vacc86/2(din)
  c_to_ri43/2 -> addr_bram_vacc87/2(din)
  [ant_11] -> cmult49/2(b)
  [ant_3] -> cmult49/1(a)
  [new_acc] -> addr_bram_vacc87/1(new_acc), addr_bram_vacc86/1(new_acc), addr_bram_vacc85/1(new_acc), addr_bram_vacc84/1(new_acc), addr_bram_vacc83/1(new_acc), addr_bram_vacc82/1(new_acc), addr_bram_vacc81/1(new_acc), addr_bram_vacc80/1(new_acc), addr_bram_vacc79/1(new_acc), addr_bram_vacc78/1(new_acc), addr_bram_vacc77/1(new_acc), addr_bram_vacc76/1(new_acc), addr_bram_vacc75/1(new_acc), addr_bram_vacc74/1(new_acc), addr_bram_vacc73/1(new_acc), addr_bram_vacc72/1(new_acc)
  cmult50/1(ab) -> c_to_ri44/1
  addr_bram_vacc88/1(addr) -> cc_a4_a5_real/1(addr)
  addr_bram_vacc88/2(dout) -> cc_a4_a5_real/2(data_in)
  addr_bram_vacc88/3(we) -> cc_a4_a5_real/3(we)
  cc_a4_a5_real/1(data_out) -> Terminator92/1
  addr_bram_vacc89/1(addr) -> cc_a4_a5_imag/1(addr)
  addr_bram_vacc89/2(dout) -> cc_a4_a5_imag/2(data_in)
  addr_bram_vacc89/3(we) -> cc_a4_a5_imag/3(we)
  cc_a4_a5_imag/1(data_out) -> Terminator93/1
  c_to_ri44/1 -> addr_bram_vacc88/2(din)
  c_to_ri44/2 -> addr_bram_vacc89/2(din)
  [ant_5] -> cmult50/2(b)
  [ant_4] -> cmult50/1(a)
  cmult51/1(ab) -> c_to_ri45/1
  addr_bram_vacc90/1(addr) -> cc_a4_a6_real/1(addr)
  addr_bram_vacc90/2(dout) -> cc_a4_a6_real/2(data_in)
  addr_bram_vacc90/3(we) -> cc_a4_a6_real/3(we)
  cc_a4_a6_real/1(data_out) -> Terminator94/1
  addr_bram_vacc91/1(addr) -> cc_a4_a6_imag/1(addr)
  addr_bram_vacc91/2(dout) -> cc_a4_a6_imag/2(data_in)
  addr_bram_vacc91/3(we) -> cc_a4_a6_imag/3(we)
  cc_a4_a6_imag/1(data_out) -> Terminator95/1
  c_to_ri45/1 -> addr_bram_vacc90/2(din)
  c_to_ri45/2 -> addr_bram_vacc91/2(din)
  [ant_6] -> cmult51/2(b)
  [ant_4] -> cmult51/1(a)
  cmult52/1(ab) -> c_to_ri47/1
  addr_bram_vacc92/1(addr) -> cc_a4_a7_real/1(addr)
  addr_bram_vacc92/2(dout) -> cc_a4_a7_real/2(data_in)
  addr_bram_vacc92/3(we) -> cc_a4_a7_real/3(we)
  cc_a4_a7_real/1(data_out) -> Terminator96/1
  addr_bram_vacc93/1(addr) -> cc_a4_a7_imag/1(addr)
  addr_bram_vacc93/2(dout) -> cc_a4_a7_imag/2(data_in)
  addr_bram_vacc93/3(we) -> cc_a4_a7_imag/3(we)
  cc_a4_a7_imag/1(data_out) -> Terminator97/1
  c_to_ri47/1 -> addr_bram_vacc92/2(din)
  c_to_ri47/2 -> addr_bram_vacc93/2(din)
  [ant_7] -> cmult52/2(b)
  [ant_4] -> cmult52/1(a)
  cmult53/1(ab) -> c_to_ri48/1
  addr_bram_vacc94/1(addr) -> cc_a4_a8_real/1(addr)
  addr_bram_vacc94/2(dout) -> cc_a4_a8_real/2(data_in)
  addr_bram_vacc94/3(we) -> cc_a4_a8_real/3(we)
  cc_a4_a8_real/1(data_out) -> Terminator98/1
  addr_bram_vacc95/1(addr) -> cc_a4_a8_imag/1(addr)
  addr_bram_vacc95/2(dout) -> cc_a4_a8_imag/2(data_in)
  addr_bram_vacc95/3(we) -> cc_a4_a8_imag/3(we)
  cc_a4_a8_imag/1(data_out) -> Terminator99/1
  c_to_ri48/1 -> addr_bram_vacc94/2(din)
  c_to_ri48/2 -> addr_bram_vacc95/2(din)
  [ant_8] -> cmult53/2(b)
  [ant_4] -> cmult53/1(a)
  cmult54/1(ab) -> c_to_ri49/1
  addr_bram_vacc96/1(addr) -> cc_a4_a9_real/1(addr)
  addr_bram_vacc96/2(dout) -> cc_a4_a9_real/2(data_in)
  addr_bram_vacc96/3(we) -> cc_a4_a9_real/3(we)
  cc_a4_a9_real/1(data_out) -> Terminator100/1
  addr_bram_vacc97/1(addr) -> cc_a4_a9_imag/1(addr)
  addr_bram_vacc97/2(dout) -> cc_a4_a9_imag/2(data_in)
  addr_bram_vacc97/3(we) -> cc_a4_a9_imag/3(we)
  cc_a4_a9_imag/1(data_out) -> Terminator101/1
  c_to_ri49/1 -> addr_bram_vacc96/2(din)
  c_to_ri49/2 -> addr_bram_vacc97/2(din)
  [ant_9] -> cmult54/2(b)
  [ant_4] -> cmult54/1(a)
  cmult55/1(ab) -> c_to_ri50/1
  addr_bram_vacc98/1(addr) -> cc_a4_a10_real/1(addr)
  addr_bram_vacc98/2(dout) -> cc_a4_a10_real/2(data_in)
  addr_bram_vacc98/3(we) -> cc_a4_a10_real/3(we)
  cc_a4_a10_real/1(data_out) -> Terminator102/1
  addr_bram_vacc99/1(addr) -> cc_a4_a10_imag/1(addr)
  addr_bram_vacc99/2(dout) -> cc_a4_a10_imag/2(data_in)
  addr_bram_vacc99/3(we) -> cc_a4_a10_imag/3(we)
  cc_a4_a10_imag/1(data_out) -> Terminator103/1
  c_to_ri50/1 -> addr_bram_vacc98/2(din)
  c_to_ri50/2 -> addr_bram_vacc99/2(din)
  [ant_10] -> cmult55/2(b)
  [ant_4] -> cmult55/1(a)
  cmult56/1(ab) -> c_to_ri51/1
  addr_bram_vacc100/1(addr) -> cc_a4_a11_real/1(addr)
  addr_bram_vacc100/2(dout) -> cc_a4_a11_real/2(data_in)
  addr_bram_vacc100/3(we) -> cc_a4_a11_real/3(we)
  cc_a4_a11_real/1(data_out) -> Terminator104/1
  addr_bram_vacc101/1(addr) -> cc_a4_a11_imag/1(addr)
  addr_bram_vacc101/2(dout) -> cc_a4_a11_imag/2(data_in)
  addr_bram_vacc101/3(we) -> cc_a4_a11_imag/3(we)
  cc_a4_a11_imag/1(data_out) -> Terminator105/1
  c_to_ri51/1 -> addr_bram_vacc100/2(din)
  c_to_ri51/2 -> addr_bram_vacc101/2(din)
  [ant_11] -> cmult56/2(b)
  [ant_4] -> cmult56/1(a)
  [new_acc] -> addr_bram_vacc93/1(new_acc), addr_bram_vacc94/1(new_acc), addr_bram_vacc101/1(new_acc), addr_bram_vacc100/1(new_acc), addr_bram_vacc99/1(new_acc), addr_bram_vacc98/1(new_acc), addr_bram_vacc97/1(new_acc), addr_bram_vacc96/1(new_acc), addr_bram_vacc95/1(new_acc), addr_bram_vacc92/1(new_acc), addr_bram_vacc91/1(new_acc), addr_bram_vacc90/1(new_acc), addr_bram_vacc89/1(new_acc), addr_bram_vacc88/1(new_acc)
  cmult57/1(ab) -> c_to_ri52/1
  addr_bram_vacc102/1(addr) -> cc_a5_a6_real/1(addr)
  addr_bram_vacc102/2(dout) -> cc_a5_a6_real/2(data_in)
  addr_bram_vacc102/3(we) -> cc_a5_a6_real/3(we)
  cc_a5_a6_real/1(data_out) -> Terminator106/1
  addr_bram_vacc103/1(addr) -> cc_a5_a6_imag/1(addr)
  addr_bram_vacc103/2(dout) -> cc_a5_a6_imag/2(data_in)
  addr_bram_vacc103/3(we) -> cc_a5_a6_imag/3(we)
  cc_a5_a6_imag/1(data_out) -> Terminator107/1
  c_to_ri52/1 -> addr_bram_vacc102/2(din)
  c_to_ri52/2 -> addr_bram_vacc103/2(din)
  [ant_6] -> cmult57/2(b)
  [ant_5] -> cmult57/1(a)
  cmult58/1(ab) -> c_to_ri53/1
  addr_bram_vacc104/1(addr) -> cc_a5_a7_real/1(addr)
  addr_bram_vacc104/2(dout) -> cc_a5_a7_real/2(data_in)
  addr_bram_vacc104/3(we) -> cc_a5_a7_real/3(we)
  cc_a5_a7_real/1(data_out) -> Terminator108/1
  addr_bram_vacc105/1(addr) -> cc_a5_a7_imag/1(addr)
  addr_bram_vacc105/2(dout) -> cc_a5_a7_imag/2(data_in)
  addr_bram_vacc105/3(we) -> cc_a5_a7_imag/3(we)
  cc_a5_a7_imag/1(data_out) -> Terminator109/1
  c_to_ri53/1 -> addr_bram_vacc104/2(din)
  c_to_ri53/2 -> addr_bram_vacc105/2(din)
  [ant_7] -> cmult58/2(b)
  [ant_5] -> cmult58/1(a)
  cmult59/1(ab) -> c_to_ri54/1
  addr_bram_vacc106/1(addr) -> cc_a5_a8_real/1(addr)
  addr_bram_vacc106/2(dout) -> cc_a5_a8_real/2(data_in)
  addr_bram_vacc106/3(we) -> cc_a5_a8_real/3(we)
  cc_a5_a8_real/1(data_out) -> Terminator110/1
  addr_bram_vacc107/1(addr) -> cc_a5_a8_imag/1(addr)
  addr_bram_vacc107/2(dout) -> cc_a5_a8_imag/2(data_in)
  addr_bram_vacc107/3(we) -> cc_a5_a8_imag/3(we)
  cc_a5_a8_imag/1(data_out) -> Terminator111/1
  c_to_ri54/1 -> addr_bram_vacc106/2(din)
  c_to_ri54/2 -> addr_bram_vacc107/2(din)
  [ant_8] -> cmult59/2(b)
  [ant_5] -> cmult59/1(a)
  cmult60/1(ab) -> c_to_ri55/1
  addr_bram_vacc108/1(addr) -> cc_a5_a9_real/1(addr)
  addr_bram_vacc108/2(dout) -> cc_a5_a9_real/2(data_in)
  addr_bram_vacc108/3(we) -> cc_a5_a9_real/3(we)
  cc_a5_a9_real/1(data_out) -> Terminator112/1
  addr_bram_vacc109/1(addr) -> cc_a5_a9_imag/1(addr)
  addr_bram_vacc109/2(dout) -> cc_a5_a9_imag/2(data_in)
  addr_bram_vacc109/3(we) -> cc_a5_a9_imag/3(we)
  cc_a5_a9_imag/1(data_out) -> Terminator113/1
  c_to_ri55/1 -> addr_bram_vacc108/2(din)
  c_to_ri55/2 -> addr_bram_vacc109/2(din)
  [ant_9] -> cmult60/2(b)
  [ant_5] -> cmult60/1(a)
  cmult61/1(ab) -> c_to_ri56/1
  addr_bram_vacc110/1(addr) -> cc_a5_a10_real/1(addr)
  addr_bram_vacc110/2(dout) -> cc_a5_a10_real/2(data_in)
  addr_bram_vacc110/3(we) -> cc_a5_a10_real/3(we)
  cc_a5_a10_real/1(data_out) -> Terminator114/1
  addr_bram_vacc111/1(addr) -> cc_a5_a10_imag/1(addr)
  addr_bram_vacc111/2(dout) -> cc_a5_a10_imag/2(data_in)
  addr_bram_vacc111/3(we) -> cc_a5_a10_imag/3(we)
  cc_a5_a10_imag/1(data_out) -> Terminator115/1
  c_to_ri56/1 -> addr_bram_vacc110/2(din)
  c_to_ri56/2 -> addr_bram_vacc111/2(din)
  [ant_10] -> cmult61/2(b)
  [ant_5] -> cmult61/1(a)
  cmult62/1(ab) -> c_to_ri58/1
  addr_bram_vacc112/1(addr) -> cc_a5_a11_real/1(addr)
  addr_bram_vacc112/2(dout) -> cc_a5_a11_real/2(data_in)
  addr_bram_vacc112/3(we) -> cc_a5_a11_real/3(we)
  cc_a5_a11_real/1(data_out) -> Terminator116/1
  addr_bram_vacc113/1(addr) -> cc_a5_a11_imag/1(addr)
  addr_bram_vacc113/2(dout) -> cc_a5_a11_imag/2(data_in)
  addr_bram_vacc113/3(we) -> cc_a5_a11_imag/3(we)
  cc_a5_a11_imag/1(data_out) -> Terminator117/1
  c_to_ri58/1 -> addr_bram_vacc112/2(din)
  c_to_ri58/2 -> addr_bram_vacc113/2(din)
  [ant_11] -> cmult62/2(b)
  [ant_5] -> cmult62/1(a)
  [new_acc] -> addr_bram_vacc113/1(new_acc), addr_bram_vacc112/1(new_acc), addr_bram_vacc111/1(new_acc), addr_bram_vacc110/1(new_acc), addr_bram_vacc109/1(new_acc), addr_bram_vacc108/1(new_acc), addr_bram_vacc107/1(new_acc), addr_bram_vacc106/1(new_acc), addr_bram_vacc105/1(new_acc), addr_bram_vacc104/1(new_acc), addr_bram_vacc103/1(new_acc), addr_bram_vacc102/1(new_acc)
  cmult63/1(ab) -> c_to_ri59/1
  addr_bram_vacc114/1(addr) -> cc_a6_a7_real/1(addr)
  addr_bram_vacc114/2(dout) -> cc_a6_a7_real/2(data_in)
  addr_bram_vacc114/3(we) -> cc_a6_a7_real/3(we)
  cc_a6_a7_real/1(data_out) -> Terminator118/1
  addr_bram_vacc115/1(addr) -> cc_a6_a7_imag/1(addr)
  addr_bram_vacc115/2(dout) -> cc_a6_a7_imag/2(data_in)
  addr_bram_vacc115/3(we) -> cc_a6_a7_imag/3(we)
  cc_a6_a7_imag/1(data_out) -> Terminator119/1
  c_to_ri59/1 -> addr_bram_vacc114/2(din)
  c_to_ri59/2 -> addr_bram_vacc115/2(din)
  [ant_7] -> cmult63/2(b)
  [ant_6] -> cmult63/1(a)
  cmult64/1(ab) -> c_to_ri60/1
  addr_bram_vacc116/1(addr) -> cc_a6_a8_real/1(addr)
  addr_bram_vacc116/2(dout) -> cc_a6_a8_real/2(data_in)
  addr_bram_vacc116/3(we) -> cc_a6_a8_real/3(we)
  cc_a6_a8_real/1(data_out) -> Terminator120/1
  addr_bram_vacc117/1(addr) -> cc_a6_a8_imag/1(addr)
  addr_bram_vacc117/2(dout) -> cc_a6_a8_imag/2(data_in)
  addr_bram_vacc117/3(we) -> cc_a6_a8_imag/3(we)
  cc_a6_a8_imag/1(data_out) -> Terminator121/1
  c_to_ri60/1 -> addr_bram_vacc116/2(din)
  c_to_ri60/2 -> addr_bram_vacc117/2(din)
  [ant_8] -> cmult64/2(b)
  [ant_6] -> cmult64/1(a)
  cmult65/1(ab) -> c_to_ri61/1
  addr_bram_vacc118/1(addr) -> cc_a6_a9_real/1(addr)
  addr_bram_vacc118/2(dout) -> cc_a6_a9_real/2(data_in)
  addr_bram_vacc118/3(we) -> cc_a6_a9_real/3(we)
  cc_a6_a9_real/1(data_out) -> Terminator122/1
  addr_bram_vacc119/1(addr) -> cc_a6_a9_imag/1(addr)
  addr_bram_vacc119/2(dout) -> cc_a6_a9_imag/2(data_in)
  addr_bram_vacc119/3(we) -> cc_a6_a9_imag/3(we)
  cc_a6_a9_imag/1(data_out) -> Terminator123/1
  c_to_ri61/1 -> addr_bram_vacc118/2(din)
  c_to_ri61/2 -> addr_bram_vacc119/2(din)
  [ant_9] -> cmult65/2(b)
  [ant_6] -> cmult65/1(a)
  cmult66/1(ab) -> c_to_ri62/1
  addr_bram_vacc120/1(addr) -> cc_a6_a10_real/1(addr)
  addr_bram_vacc120/2(dout) -> cc_a6_a10_real/2(data_in)
  addr_bram_vacc120/3(we) -> cc_a6_a10_real/3(we)
  cc_a6_a10_real/1(data_out) -> Terminator124/1
  addr_bram_vacc121/1(addr) -> cc_a6_a10_imag/1(addr)
  addr_bram_vacc121/2(dout) -> cc_a6_a10_imag/2(data_in)
  addr_bram_vacc121/3(we) -> cc_a6_a10_imag/3(we)
  cc_a6_a10_imag/1(data_out) -> Terminator125/1
  c_to_ri62/1 -> addr_bram_vacc120/2(din)
  c_to_ri62/2 -> addr_bram_vacc121/2(din)
  [ant_10] -> cmult66/2(b)
  [ant_6] -> cmult66/1(a)
  cmult67/1(ab) -> c_to_ri63/1
  addr_bram_vacc122/1(addr) -> cc_a6_a11_real/1(addr)
  addr_bram_vacc122/2(dout) -> cc_a6_a11_real/2(data_in)
  addr_bram_vacc122/3(we) -> cc_a6_a11_real/3(we)
  cc_a6_a11_real/1(data_out) -> Terminator126/1
  addr_bram_vacc123/1(addr) -> cc_a6_a11_imag/1(addr)
  addr_bram_vacc123/2(dout) -> cc_a6_a11_imag/2(data_in)
  addr_bram_vacc123/3(we) -> cc_a6_a11_imag/3(we)
  cc_a6_a11_imag/1(data_out) -> Terminator127/1
  c_to_ri63/1 -> addr_bram_vacc122/2(din)
  c_to_ri63/2 -> addr_bram_vacc123/2(din)
  [ant_11] -> cmult67/2(b)
  [ant_6] -> cmult67/1(a)
  [new_acc] -> addr_bram_vacc123/1(new_acc), addr_bram_vacc122/1(new_acc), addr_bram_vacc121/1(new_acc), addr_bram_vacc120/1(new_acc), addr_bram_vacc119/1(new_acc), addr_bram_vacc118/1(new_acc), addr_bram_vacc117/1(new_acc), addr_bram_vacc116/1(new_acc), addr_bram_vacc115/1(new_acc), addr_bram_vacc114/1(new_acc)
  cmult68/1(ab) -> c_to_ri64/1
  addr_bram_vacc124/1(addr) -> cc_a7_a8_real/1(addr)
  addr_bram_vacc124/2(dout) -> cc_a7_a8_real/2(data_in)
  addr_bram_vacc124/3(we) -> cc_a7_a8_real/3(we)
  cc_a7_a8_real/1(data_out) -> Terminator128/1
  addr_bram_vacc125/1(addr) -> cc_a7_a8_imag/1(addr)
  addr_bram_vacc125/2(dout) -> cc_a7_a8_imag/2(data_in)
  addr_bram_vacc125/3(we) -> cc_a7_a8_imag/3(we)
  cc_a7_a8_imag/1(data_out) -> Terminator129/1
  c_to_ri64/1 -> addr_bram_vacc124/2(din)
  c_to_ri64/2 -> addr_bram_vacc125/2(din)
  [ant_8] -> cmult68/2(b)
  [ant_7] -> cmult68/1(a)
  cmult69/1(ab) -> c_to_ri65/1
  addr_bram_vacc126/1(addr) -> cc_a7_a9_real/1(addr)
  addr_bram_vacc126/2(dout) -> cc_a7_a9_real/2(data_in)
  addr_bram_vacc126/3(we) -> cc_a7_a9_real/3(we)
  cc_a7_a9_real/1(data_out) -> Terminator130/1
  addr_bram_vacc127/1(addr) -> cc_a7_a9_imag/1(addr)
  addr_bram_vacc127/2(dout) -> cc_a7_a9_imag/2(data_in)
  addr_bram_vacc127/3(we) -> cc_a7_a9_imag/3(we)
  cc_a7_a9_imag/1(data_out) -> Terminator131/1
  c_to_ri65/1 -> addr_bram_vacc126/2(din)
  c_to_ri65/2 -> addr_bram_vacc127/2(din)
  [ant_9] -> cmult69/2(b)
  [ant_7] -> cmult69/1(a)
  cmult70/1(ab) -> c_to_ri66/1
  addr_bram_vacc128/1(addr) -> cc_a7_a10_real/1(addr)
  addr_bram_vacc128/2(dout) -> cc_a7_a10_real/2(data_in)
  addr_bram_vacc128/3(we) -> cc_a7_a10_real/3(we)
  cc_a7_a10_real/1(data_out) -> Terminator132/1
  addr_bram_vacc129/1(addr) -> cc_a7_a10_imag/1(addr)
  addr_bram_vacc129/2(dout) -> cc_a7_a10_imag/2(data_in)
  addr_bram_vacc129/3(we) -> cc_a7_a10_imag/3(we)
  cc_a7_a10_imag/1(data_out) -> Terminator133/1
  c_to_ri66/1 -> addr_bram_vacc128/2(din)
  c_to_ri66/2 -> addr_bram_vacc129/2(din)
  [ant_10] -> cmult70/2(b)
  [ant_7] -> cmult70/1(a)
  cmult71/1(ab) -> c_to_ri67/1
  addr_bram_vacc130/1(addr) -> cc_a7_a11_real/1(addr)
  addr_bram_vacc130/2(dout) -> cc_a7_a11_real/2(data_in)
  addr_bram_vacc130/3(we) -> cc_a7_a11_real/3(we)
  cc_a7_a11_real/1(data_out) -> Terminator134/1
  addr_bram_vacc131/1(addr) -> cc_a7_a11_imag/1(addr)
  addr_bram_vacc131/2(dout) -> cc_a7_a11_imag/2(data_in)
  addr_bram_vacc131/3(we) -> cc_a7_a11_imag/3(we)
  cc_a7_a11_imag/1(data_out) -> Terminator135/1
  c_to_ri67/1 -> addr_bram_vacc130/2(din)
  c_to_ri67/2 -> addr_bram_vacc131/2(din)
  [ant_11] -> cmult71/2(b)
  [ant_7] -> cmult71/1(a)
  [new_acc] -> addr_bram_vacc131/1(new_acc), addr_bram_vacc130/1(new_acc), addr_bram_vacc129/1(new_acc), addr_bram_vacc128/1(new_acc), addr_bram_vacc127/1(new_acc), addr_bram_vacc126/1(new_acc), addr_bram_vacc125/1(new_acc), addr_bram_vacc124/1(new_acc)
  cmult72/1(ab) -> c_to_ri69/1
  addr_bram_vacc132/1(addr) -> cc_a8_a9_real/1(addr)
  addr_bram_vacc132/2(dout) -> cc_a8_a9_real/2(data_in)
  addr_bram_vacc132/3(we) -> cc_a8_a9_real/3(we)
  cc_a8_a9_real/1(data_out) -> Terminator136/1
  addr_bram_vacc133/1(addr) -> cc_a8_a9_imag/1(addr)
  addr_bram_vacc133/2(dout) -> cc_a8_a9_imag/2(data_in)
  addr_bram_vacc133/3(we) -> cc_a8_a9_imag/3(we)
  cc_a8_a9_imag/1(data_out) -> Terminator137/1
  c_to_ri69/1 -> addr_bram_vacc132/2(din)
  c_to_ri69/2 -> addr_bram_vacc133/2(din)
  [ant_9] -> cmult72/2(b)
  [ant_8] -> cmult72/1(a)
  cmult73/1(ab) -> c_to_ri70/1
  addr_bram_vacc134/1(addr) -> cc_a8_a10_real/1(addr)
  addr_bram_vacc134/2(dout) -> cc_a8_a10_real/2(data_in)
  addr_bram_vacc134/3(we) -> cc_a8_a10_real/3(we)
  cc_a8_a10_real/1(data_out) -> Terminator138/1
  addr_bram_vacc135/1(addr) -> cc_a8_a10_imag/1(addr)
  addr_bram_vacc135/2(dout) -> cc_a8_a10_imag/2(data_in)
  addr_bram_vacc135/3(we) -> cc_a8_a10_imag/3(we)
  cc_a8_a10_imag/1(data_out) -> Terminator139/1
  c_to_ri70/1 -> addr_bram_vacc134/2(din)
  c_to_ri70/2 -> addr_bram_vacc135/2(din)
  [ant_10] -> cmult73/2(b)
  [ant_8] -> cmult73/1(a)
  cmult74/1(ab) -> c_to_ri71/1
  addr_bram_vacc136/1(addr) -> cc_a8_a11_real/1(addr)
  addr_bram_vacc136/2(dout) -> cc_a8_a11_real/2(data_in)
  addr_bram_vacc136/3(we) -> cc_a8_a11_real/3(we)
  cc_a8_a11_real/1(data_out) -> Terminator140/1
  addr_bram_vacc137/1(addr) -> cc_a8_a11_imag/1(addr)
  addr_bram_vacc137/2(dout) -> cc_a8_a11_imag/2(data_in)
  addr_bram_vacc137/3(we) -> cc_a8_a11_imag/3(we)
  cc_a8_a11_imag/1(data_out) -> Terminator141/1
  c_to_ri71/1 -> addr_bram_vacc136/2(din)
  c_to_ri71/2 -> addr_bram_vacc137/2(din)
  [ant_11] -> cmult74/2(b)
  [ant_8] -> cmult74/1(a)
  [new_acc] -> addr_bram_vacc137/1(new_acc), addr_bram_vacc136/1(new_acc), addr_bram_vacc135/1(new_acc), addr_bram_vacc134/1(new_acc), addr_bram_vacc133/1(new_acc), addr_bram_vacc132/1(new_acc)
  cmult75/1(ab) -> c_to_ri72/1
  addr_bram_vacc138/1(addr) -> cc_a9_a10_real/1(addr)
  addr_bram_vacc138/2(dout) -> cc_a9_a10_real/2(data_in)
  addr_bram_vacc138/3(we) -> cc_a9_a10_real/3(we)
  cc_a9_a10_real/1(data_out) -> Terminator142/1
  addr_bram_vacc139/1(addr) -> cc_a9_a10_imag/1(addr)
  addr_bram_vacc139/2(dout) -> cc_a9_a10_imag/2(data_in)
  addr_bram_vacc139/3(we) -> cc_a9_a10_imag/3(we)
  cc_a9_a10_imag/1(data_out) -> Terminator143/1
  c_to_ri72/1 -> addr_bram_vacc138/2(din)
  c_to_ri72/2 -> addr_bram_vacc139/2(din)
  [ant_10] -> cmult75/2(b)
  [ant_9] -> cmult75/1(a)
  cmult76/1(ab) -> c_to_ri73/1
  addr_bram_vacc140/1(addr) -> cc_a9_a11_real/1(addr)
  addr_bram_vacc140/2(dout) -> cc_a9_a11_real/2(data_in)
  addr_bram_vacc140/3(we) -> cc_a9_a11_real/3(we)
  cc_a9_a11_real/1(data_out) -> Terminator144/1
  addr_bram_vacc141/1(addr) -> cc_a9_a11_imag/1(addr)
  addr_bram_vacc141/2(dout) -> cc_a9_a11_imag/2(data_in)
  addr_bram_vacc141/3(we) -> cc_a9_a11_imag/3(we)
  cc_a9_a11_imag/1(data_out) -> Terminator145/1
  c_to_ri73/1 -> addr_bram_vacc140/2(din)
  c_to_ri73/2 -> addr_bram_vacc141/2(din)
  [ant_11] -> cmult76/2(b)
  [ant_9] -> cmult76/1(a)
  [new_acc] -> addr_bram_vacc141/1(new_acc), addr_bram_vacc140/1(new_acc), addr_bram_vacc139/1(new_acc), addr_bram_vacc138/1(new_acc)
  cmult77/1(ab) -> c_to_ri74/1
  addr_bram_vacc142/1(addr) -> cc_a10_a11_real/1(addr)
  addr_bram_vacc142/2(dout) -> cc_a10_a11_real/2(data_in)
  addr_bram_vacc142/3(we) -> cc_a10_a11_real/3(we)
  cc_a10_a11_real/1(data_out) -> Terminator146/1
  addr_bram_vacc143/1(addr) -> cc_a10_a11_imag/1(addr)
  addr_bram_vacc143/2(dout) -> cc_a10_a11_imag/2(data_in)
  addr_bram_vacc143/3(we) -> cc_a10_a11_imag/3(we)
  cc_a10_a11_imag/1(data_out) -> Terminator147/1
  c_to_ri74/1 -> addr_bram_vacc142/2(din)
  c_to_ri74/2 -> addr_bram_vacc143/2(din)
  [ant_11] -> cmult77/2(b)
  [ant_10] -> cmult77/1(a)
  [new_acc] -> addr_bram_vacc143/1(new_acc), addr_bram_vacc142/1(new_acc)
